simulink model "TosAeb"  (R2021a)
settings: Solver=FixedStepDiscrete  StartTime=0.0  StopTime=inf  FixedStep=0.01  RelTol=1e-3
undefined symbols (not defined in the file or in its folder): ACCModSts, AccSts1, AEB_ObjectMovingOrientation, AEB_ObjectMovingState, CN_BTCActive, CN_CurrentGearR, CN_CurrentGearR_DHT, CN_PTCActive, CN_VDCActive, CN_VSC_EspOff, CN_VSC_FCW_AEB_Error, CN_VSC_FCW_AEB_NoDispay, CN_VSC_FCW_AEB_PerfmDgdtn, CN_VSC_FCW_AEB_TempUnAvlbl, CN_VSC_VehStandstill, CNaccelAvoidMax, CNaccelAvoidMin, CNbrakePedalPress, CNcomfortStrLeftAccelMax, CNcomfortStrLeftAccelMin, CNcomfortStrRightAccelMax, CNcomfortStrRightAccelMin, CNDrvDoorOpen, CNDrvModeEco, CNDrvModeNorm, CNDrvModeOther, CNDrvModeSport, CNDrvSBRreminder, CNNull, CNobjInvalidID, CNPassDoorOpen, CNPassSBRreminder, CNPEBSAccelNegInf, CNPEBSAccelNull, CNPEBSTimeInf, CNPEBSTimeNull, CNSTOPMAX, CNSTOPNULL, CNsubjWidth, CNSwtOFF, CNSwtON, CNTTCMAX, CNTTCNULL, CNVehConfigAEBCycNone, CNVehConfigAEBNone, CNVehConfigAEBPedNone, CNVehConfigFCWNone, CNVehConfigJANone, CollisionSeverity, FALSE, FCWSnvty, JACycObjCalcInfoDefaultParams, JAPedObjCalcInfoDefaultParams, JAVehObjCalcInfoDefaultParams, latCycObjCalcInfoDefaultParams, latPedObjCalcInfoDefaultParams, longObjCalcInfoDefaultParams, ModSts, movingStatus, ObjectType ... (+1112)
libraries: simulink x3031, AEBSFunctionLib x50, Ta_Library x33, AEBSStateMachineLib x23, Mylibrary x12, simulink_extras x7, ObjSelect_lib x3, dspstat3 x2
(part 29 of 102)

== system TosAeb/FcwAebJa_Step_sys/ALG_AEBSystem/ALG_AEBController/AEB_FuncImpManagementApp/FuncManeuArbCalc/brkFuncManeuArbCalc/axTgtReqValueCalc1 ==
  AEBModStsFuncImpArb : Inport
  FCTB_BrkgReqVal : Inport  Port=2
  VehicleStandstill : Inport  Port=3
  VSC_VehStandstill : Inport  Port=4
  subjVel : Inport  Port=5
  subjLongAccel : Inport  Port=6
  ObjectMovingState : Inport  Port=7
  vx : Inport  Port=8
  ax : Inport  Port=9
  dxFrontBumper : Inport  Port=10
  axAbsObj : Inport  Port=11  OutDataTypeStr=single
  vxAbsObj : Inport  Port=12
  AebfullTriggerInfoCalc : Subsystem
  Bus Creator : BusCreator  Inputs=12
  Bus Selector : BusSelector  OutputSignals=AEBModStsFuncImpArb.AEBfullFuncImpArb
  Bus Selector1 : BusSelector  OutputSignals=AEBModStsFuncImpArb.FCTBFuncImpArb,VehicleStandstill,subjVel,FCTB_BrkgReqVal
  Bus Selector2 : BusSelector  OutputSignals=AEBModStsFuncImpArb.AEBfullFuncImpArb,VSC_VehStandstill,subjVel,dxFrontBumper,vx,vxAbsObj,axAbsObj,ax,ObjectMovingState
  Bus Selector3 : BusSelector  OutputSignals=AEBModStsFuncImpArb,subjVel,FCTB_BrkgReqVal,ObjectMovingState
  Bus Selector4 : BusSelector  OutputSignals=VSC_VehStandstill,subjVel,vx,subjLongAccel
  Compare To Constant : simulink/Logic and Bit Operations/Compare To Constant  relop===  const=ModSts.ActiveState  OutDataTypeStr=boolean
  Constant : Constant  Value=P_AEB_FIM_BrkControllerConfig
  Fctb_IsNeedBrakeCalc : Subsystem
  If : If  IfExpression=u1
  Logical Operator : Logic  AllPortsSameDT=off  OutDataTypeStr=boolean
  Merge : Merge
  Unit Delay : UnitDelay  SampleTime=-1  HasFrameUpgradeWarning=on
  axTgtReqValueCalc4AebControl : Subsystem
  axTgtReqValueCalc4AebFull : Subsystem
  FctbIsNeedBrake : Outport
  axTgtReqValue : Outport  Port=2
  wires:
  Bus Selector3/4 -> axTgtReqValueCalc4AebFull/5(ObjectMovingState)  "<ObjectMovingState>"
  Logical Operator/1 -> If/1
  Constant/1 -> Logical Operator/2
  Compare To Constant/1 -> Logical Operator/1
  [axTgtReqValueK1] -> axTgtReqValueCalc4AebControl/9(axTgtReqValueK1)
  Bus Selector4/4 -> axTgtReqValueCalc4AebControl/8(subjLongAccel)  "<subjLongAccel>"
  Bus Selector4/3 -> axTgtReqValueCalc4AebControl/7(vx)  "<vx>"
  AebfullTriggerInfoCalc/4(axAbsObjFilt) -> axTgtReqValueCalc4AebControl/4(axAbsObj)
  Bus Selector4/2 -> axTgtReqValueCalc4AebControl/6(subjVel)  "<subjVel>"
  Bus Selector3/3 -> axTgtReqValueCalc4AebFull/4(FCTB_BrkgReqVal)  "<FCTB_BrkgReqVal>"
  Bus Selector3/2 -> axTgtReqValueCalc4AebFull/3(subjVel)  "<subjVel>"
  Bus Selector3/1 -> axTgtReqValueCalc4AebFull/2(AEBModStsFuncImpArb)  "<AEBModStsFuncImpArb>"
  Unit Delay/1 -> axTgtReqValueCalc4AebFull/6(axTgtReqValueK1), [axTgtReqValueK1]
  axTgtReqValueCalc4AebFull/1(axTgtReqValue) -> Merge/2
  axTgtReqValueCalc4AebControl/1(axTgtReqValue) -> Merge/1
  [AebFullBrkInActive] -> axTgtReqValueCalc4AebFull/Action
  If/2 -> [AebFullBrkInActive]
  Bus Selector2/9 -> AebfullTriggerInfoCalc/9(ObjectMovingState)  "<ObjectMovingState>"
  Bus Selector2/8 -> AebfullTriggerInfoCalc/8(ax)  "<ax>"
  Bus Selector2/5 -> AebfullTriggerInfoCalc/5(vx)  "<vx>"
  Bus Selector2/4 -> AebfullTriggerInfoCalc/4(dxFrontBumper)  "<dxFrontBumper>"
  Bus Selector2/3 -> AebfullTriggerInfoCalc/3(subjVel)  "<subjVel>"
  Bus Selector2/1 -> AebfullTriggerInfoCalc/1(AEBfullFuncImpArb)  "<AEBfullFuncImpArb>"
  Bus Selector1/4 -> Fctb_IsNeedBrakeCalc/4(FCTB_BrkgReqVal)  "<FCTB_BrkgReqVal>"
  Bus Selector1/3 -> Fctb_IsNeedBrakeCalc/3(subjVel)  "<subjVel>"
  Bus Selector1/2 -> Fctb_IsNeedBrakeCalc/2(VehicleStandstill)  "<VehicleStandstill>"
  Bus Selector1/1 -> Fctb_IsNeedBrakeCalc/1(FctbReq)  "<FCTBFuncImpArb>"
  subjLongAccel/1 -> Bus Creator/6  "subjLongAccel"
  Bus Creator/1 -> Bus Selector/1, Bus Selector1/1, Bus Selector3/1, Bus Selector4/1, Bus Selector2/1
  axAbsObj/1 -> Bus Creator/11  "axAbsObj"
  dxFrontBumper/1 -> Bus Creator/10  "dxFrontBumper"
  ax/1 -> Bus Creator/9  "ax"
  vx/1 -> Bus Creator/8  "vx"
  ObjectMovingState/1 -> Bus Creator/7  "ObjectMovingState"
  subjVel/1 -> Bus Creator/5  "subjVel"
  VehicleStandstill/1 -> Bus Creator/3  "VehicleStandstill"
  FCTB_BrkgReqVal/1 -> Bus Creator/2  "FCTB_BrkgReqVal"
  AEBModStsFuncImpArb/1 -> Bus Creator/1  "AEBModStsFuncImpArb"
  If/1 -> axTgtReqValueCalc4AebControl/Action
  Bus Selector/1 -> Compare To Constant/1  "<AEBfullFuncImpArb>"
  AebfullTriggerInfoCalc/3(AEB_FIM_BrakeInfo) -> axTgtReqValueCalc4AebControl/3(AEB_FIM_BrakeInfo)
  AebfullTriggerInfoCalc/2(ObjectPredicted) -> axTgtReqValueCalc4AebControl/2(ObjectPredicted)
  AebfullTriggerInfoCalc/1(AebTriggerInfo) -> axTgtReqValueCalc4AebControl/1(AebTriggerInfo)
  Fctb_IsNeedBrakeCalc/1(IsNeedBrake) -> axTgtReqValueCalc4AebFull/1(AEB_FctbIsNeedBrake), FctbIsNeedBrake/1  "AEB_FctbIsNeedBrake"
  Merge/1 -> axTgtReqValue/1, Unit Delay/1
  VSC_VehStandstill/1 -> Bus Creator/4  "VSC_VehStandstill"
  Bus Selector4/1 -> axTgtReqValueCalc4AebControl/5(VSC_VehStandstill)  "<VSC_VehStandstill>"
  vxAbsObj/1 -> Bus Creator/12  "vxAbsObj"
  Bus Selector2/6 -> AebfullTriggerInfoCalc/6(vxAbsObj)  "<vxAbsObj>"
  Bus Selector2/2 -> AebfullTriggerInfoCalc/2(VSC_VehStandstill)  "<VSC_VehStandstill>"
  Bus Selector2/7 -> AebfullTriggerInfoCalc/7(axAbsObj)  "<axAbsObj>"

== system TosAeb/FcwAebJa_Step_sys/ALG_AEBSystem/ALG_AEBController/AEB_FuncImpManagementApp/FuncManeuArbCalc/brkFuncManeuArbCalc/axTgtReqValueManeuCalc ==
  AEBTrigger : Inport
  axTgtReqValue : Inport  Port=2  OutDataTypeStr=single
  AEBQuitFlgJudge : Subsystem
  AEBTriggerManeuCalc : Stateflow chart  TreatAsAtomicUnit=on
  AEBTriggerManeuCalc1 : Stateflow chart  Commented=on  TreatAsAtomicUnit=on
  Constant : Constant  Value=Ts  OutDataTypeStr=single
  Constant1 : Constant  Value=P_AEB_FIM_BrkFuncMaxTimeQuitThres  OutDataTypeStr=single
  Constant2 : Constant  Value=P_AEB_FIM_DecelGradientQuitThres  OutDataTypeStr=single
  Unit Delay1 : UnitDelay  SampleTime=-1  HasFrameUpgradeWarning=on
  AEBTriggerManeu : Outport
  axTgtReqValueManeu : Outport  Port=2
  wires:
  Unit Delay1/1 -> AEBTriggerManeuCalc/7(axTgtReqValueManeuK1)
  AEBTriggerManeuCalc/2(axTgtReqValueManeu) -> axTgtReqValueManeu/1, Unit Delay1/1
  AEBTriggerManeuCalc/1(AEBTriggerManeu) -> AEBTriggerManeu/1
  axTgtReqValue/1 -> AEBTriggerManeuCalc/3(axTgtReqValue)
  Constant/1 -> AEBTriggerManeuCalc/4(Ts)
  Constant1/1 -> AEBTriggerManeuCalc/5(PmaxQuitTime)
  AEBQuitFlgJudge/1(AEBQuitFlg) -> AEBTriggerManeuCalc/1(AEBQuitFlg)
  AEBTrigger/1 -> AEBQuitFlgJudge/1(AEBTrigger), AEBTriggerManeuCalc/2(AEBTrigger)
  Constant2/1 -> AEBTriggerManeuCalc/6(axJerkQuit)

== system TosAeb/FcwAebJa_Step_sys/ALG_AEBSystem/ALG_AEBController/AEB_FuncImpManagementApp/FuncManeuArbCalc/brkFuncManeuArbCalc/brkManeuAborCalc ==
  AEBTrigger : Inport
  subjVel : Inport  Port=2  OutDataTypeStr=single
  EBAFuncSts : Inport  Port=3
  accelAvoid : Inport  Port=4
  ObjectMovingState : Inport  Port=5
  axAbsObj : Inport  Port=6
  AEBBA : Inport  Port=7
  AEBIB : Inport  Port=8
  vx : Inport  Port=9
  Bus Creator : BusCreator  Inputs=5
  Bus Selector : BusSelector  OutputSignals=AEBTrigger,VelReduceMaxCond,dgrCond,ESPRelAborCond,VelCond
  Bus Selector1 : BusSelector  OutputSignals=AEBTrigger,VelReduceMaxCond,dgrCond,ESPRelAborCond
  Bus Selector2 : BusSelector  OutputSignals=AEBTrigger,VelReduceMaxCond,dgrCond,ESPRelAborCond
  Constant : Constant  Value=FALSE
  Constant1 : Constant  Value=FALSE
  Constant2 : Constant  Value=FALSE
  DgrCondCalc : Subsystem
  ESPRelAborCondCalc : Subsystem
  JABrkManeuAborCalc : Subsystem
  LatBrkManeuAborCalc : Subsystem
  Terminator : Terminator
  Terminator1 : Terminator
  Terminator2 : Terminator
  VelCond : Subsystem
  VelReduceMaxCond : Subsystem
  vehBrkManeuAborCalc : Subsystem
  vehBrkManeuAbor : Outport
  latBrkManeuAbor : Outport  Port=2
  JABrkManeuAbor : Outport  Port=3
  wires:
  Bus Selector/5 -> vehBrkManeuAborCalc/5(velAborCond)  "<VelCond>"
  VelCond/1(VelCond) -> Bus Creator/3  "VelCond"
  vx/1 -> VelCond/1(vx)
  EBAFuncSts/1 -> VelReduceMaxCond/3(EBAFuncSts)
  JABrkManeuAborCalc/1(JABrkManeuAbor) -> JABrkManeuAbor/1
  LatBrkManeuAborCalc/1(latBrkManeuAbor) -> latBrkManeuAbor/1
  Constant2/1 -> JABrkManeuAborCalc/2(VelReduceMaxCond)
  Bus Selector2/2 -> Terminator2/1  "<VelReduceMaxCond>"
  Bus Selector2/1 -> JABrkManeuAborCalc/1(AEBTrigger)  "<AEBTrigger>"
  Bus Selector2/3 -> Terminator1/1  "<dgrCond>"
  Constant1/1 -> JABrkManeuAborCalc/3(dgrAborCond)
  Bus Selector2/4 -> JABrkManeuAborCalc/4(ESPRelAborCond)  "<ESPRelAborCond>"
  Bus Selector1/4 -> LatBrkManeuAborCalc/4(ESPRelAborCond)  "<ESPRelAborCond>"
  Constant/1 -> LatBrkManeuAborCalc/3(dgrAborCond)
  Bus Selector1/3 -> Terminator/1  "<dgrCond>"
  Bus Selector1/2 -> LatBrkManeuAborCalc/2(VelReduceMaxCond)  "<VelReduceMaxCond>"
  Bus Selector1/1 -> LatBrkManeuAborCalc/1(AEBTrigger)  "<AEBTrigger>"
  Bus Selector/1 -> vehBrkManeuAborCalc/1(AEBTrigger)  "<AEBTrigger>"
  Bus Selector/4 -> vehBrkManeuAborCalc/4(ESPRelAborCond)  "<ESPRelAborCond>"
  Bus Selector/2 -> vehBrkManeuAborCalc/2(VelReduceMaxCond)  "<VelReduceMaxCond>"
  Bus Selector/3 -> vehBrkManeuAborCalc/3(dgrAborCond)  "<dgrCond>"
  Bus Creator/1 -> Bus Selector1/1, Bus Selector2/1, Bus Selector/1
  ESPRelAborCondCalc/1(ESPRelAborCond) -> Bus Creator/5  "ESPRelAborCond"
  DgrCondCalc/1(dgrCond) -> Bus Creator/4  "dgrCond"
  VelReduceMaxCond/1(VelReduceMaxCond) -> Bus Creator/2  "VelReduceMaxCond"
  AEBIB/1 -> ESPRelAborCondCalc/2(AEBIB)
  AEBBA/1 -> ESPRelAborCondCalc/1(AEBBA)
  accelAvoid/1 -> DgrCondCalc/3(accelAvoid)
  ObjectMovingState/1 -> DgrCondCalc/1(ObjectMovingState), VelCond/3(ObjectMovingState)
  axAbsObj/1 -> DgrCondCalc/2(axAbsObj), VelCond/2(axAbsObj)
  vehBrkManeuAborCalc/1(vehBrkManeuAbor) -> vehBrkManeuAbor/1
  AEBTrigger/1 -> VelReduceMaxCond/1(AEBTrigger), Bus Creator/1  "AEBTrigger"
  subjVel/1 -> VelReduceMaxCond/2(subjVel)

== system TosAeb/FcwAebJa_Step_sys/ALG_AEBSystem/ALG_AEBController/AEB_JaObjCalcApp/JAPedTgtInfoCalc/JAPedCollisonAssessInfo/LatPedCollisonAssessInfoCalc ==
  vx : Inport  OutDataTypeStr=single
  vy : Inport  Port=2  OutDataTypeStr=single
  dx : Inport  Port=3  OutDataTypeStr=single
  dy : Inport  Port=4  OutDataTypeStr=single
  dxFrontBumper : Inport  Port=5  OutDataTypeStr=single
  tPredictTime : Inport  Port=6  OutDataTypeStr=single
  subjYawRate : Inport  Port=7  OutDataTypeStr=single
  subjVel1 : Inport  Port=8  OutDataTypeStr=single
  subjLongAccel : Inport  Port=9  OutDataTypeStr=single
  Action Port : ActionPort  ActionPortLabel=else
  Bus Creator : BusCreator  Inputs=4
  PedDistBrakeFullCalc : Subsystem
  Subsystem1 : Subsystem
  VelAbsTgtCorrCalc : Subsystem
  distRadical2CollsionCalc : Subsystem
  tBrakeingCalc : Subsystem  Variant=on
  JAPedCollisonAssessInfo : Outport
  wires:
  Bus Creator/1 -> JAPedCollisonAssessInfo/1
  VelAbsTgtCorrCalc/2(vyAbsTgtCorr) -> distRadical2CollsionCalc/2(vyAbsTgtCorr), Subsystem1/2(vyAbsTargetCorr)
  tPredictTime/1 -> distRadical2CollsionCalc/5(tPredictTime)
  tBrakeingCalc/1(TTB) -> Bus Creator/4  "TTB"
  subjLongAccel/1 -> PedDistBrakeFullCalc/1(subjLongAccel)
  dxFrontBumper/1 -> VelAbsTgtCorrCalc/4(dxFrontBumper)
  Subsystem1/1(TgtYawAngle) -> Bus Creator/1  "TgtYawAngle"
  subjYawRate/1 -> VelAbsTgtCorrCalc/5(subjYawRate)
  PedDistBrakeFullCalc/1(distBrake) -> Bus Creator/3  "distBraking"
  [dy] -> distRadical2CollsionCalc/4(dy)
  vy/1 -> VelAbsTgtCorrCalc/2(vy)
  dx/1 -> distRadical2CollsionCalc/3(dx)
  PedDistBrakeFullCalc/2(tBrake) -> tBrakeingCalc/1(tBarke)
  dy/1 -> [dy], VelAbsTgtCorrCalc/3(dy)
  distRadical2CollsionCalc/1(distRadical2Collsion) -> Bus Creator/2  "distRadical2Collsion"
  subjVel1/1 -> VelAbsTgtCorrCalc/6(subjVel), PedDistBrakeFullCalc/2(subjVel), tBrakeingCalc/2(subjVel)
  VelAbsTgtCorrCalc/1(vxAbsTgtCorr) -> distRadical2CollsionCalc/1(vxAbsTgtCorr), Subsystem1/1(vxAbsTargetCorr)
  vx/1 -> VelAbsTgtCorrCalc/1(vx)

== system TosAeb/FcwAebJa_Step_sys/ALG_AEBSystem/ALG_AEBController/AEB_JaObjCalcApp/JAPedTgtInfoCalc/JAPedCollisonAssessInfo/LatPedCollisonAssessInfoDefault ==
  Action Port : ActionPort  ActionPortLabel=if(u1)
  Bus Creator : BusCreator  Inputs=4
  Constant : Constant  Value=0  OutDataTypeStr=single
  Constant1 : Constant  Value=255  OutDataTypeStr=single
  Constant2 : Constant  Value=255  OutDataTypeStr=single
  Constant3 : Constant  Value=256  OutDataTypeStr=single
  JAPedCollisonAssessInfo : Outport
  wires:
  Constant2/1 -> Bus Creator/3  "distBraking"
  Constant1/1 -> Bus Creator/2  "distRadical2Collsion"
  Bus Creator/1 -> JAPedCollisonAssessInfo/1
  Constant/1 -> Bus Creator/1  "TgtYawAngle"
  Constant3/1 -> Bus Creator/4  "TTB"

== system TosAeb/FcwAebJa_Step_sys/ALG_AEBSystem/ALG_AEBController/AEB_JaObjCalcApp/JAPedTgtInfoCalc/JAPedCollisonAssessInfo/ObjIsInvalidJudge ==
  ObjectID : Inport
  Compare To Constant : simulink/Logic and Bit Operations/Compare To Constant  relop===  const=CNobjInvalidID  OutDataTypeStr=boolean
  ObjIsInvalid : Outport
  wires:
  ObjectID/1 -> Compare To Constant/1
  Compare To Constant/1 -> ObjIsInvalid/1

== system TosAeb/FcwAebJa_Step_sys/ALG_AEBSystem/ALG_AEBController/AEB_JaObjCalcApp/JAPedTgtInfoCalc/JAPedObjCalcInfoCalc/JAPedObjInfoCalc ==
  AEBJAVehObj : Inport
  subjInfo : Inport  Port=2
  Action Port : ActionPort  ActionPortLabel=else
  Bus Creator : BusCreator  Inputs=3  OutDataTypeStr=Bus: BusAEBJAPedObjCalcInfo  NonVirtualBus=on
      settings: InheritFromInputs=off
  Bus Creator1 : BusCreator  Inputs=6  OutDataTypeStr=Bus: BusAEBJAPedObjAbsInfo  NonVirtualBus=on
      settings: InheritFromInputs=off
  Bus Selector : BusSelector  OutputSignals=dx,dy,vx,ax,ObjectID,ObjectTypeCameraConfirmed,FR_ObjectID,FR_Dx,FR_Vy,FC_ObjectID,FC_Dx,FC_Vy
  Bus Selector1 : BusSelector  OutputSignals=subjLongAccel,subjYawRate,subjVel
  ObjCrossFlgCal : Subsystem
  SensorTgtInfo2AbsTgtInfoTrans : Subsystem
  "JAPedObjCalcInfo " : Outport  OutDataTypeStr=Bus: BusAEBJAPedObjCalcInfo
  wires:
  ObjCrossFlgCal/1(ObjColliInfo) -> Bus Creator/3  "BusAEBJAObjColliInfo"
  [ObjAbsInfo] -> Bus Creator/2  "BusAEBJAObjAbsInfo"
  Bus Creator1/1 -> [ObjAbsInfo], ObjCrossFlgCal/2(ObjAbsInfo)
  subjInfo/1 -> Bus Selector1/1, ObjCrossFlgCal/3(subjInfo)
  AEBJAVehObj/1 -> Bus Selector/1, Bus Creator/1, ObjCrossFlgCal/1(AEBJAPedObj)  "AEBJAPedObj"
  Bus Creator/1 -> "JAPedObjCalcInfo "/1
  SensorTgtInfo2AbsTgtInfoTrans/6(ObjectYawAngleCorr) -> Bus Creator1/6  "ObjectYawAngleCorr"
  Bus Selector/7 -> SensorTgtInfo2AbsTgtInfoTrans/7(FR_ObjectID)  "<FR_ObjectID>"
  SensorTgtInfo2AbsTgtInfoTrans/5(ObjFusQuality) -> Bus Creator1/5  "ObjFusQuality"
  SensorTgtInfo2AbsTgtInfoTrans/1(dxFrontBumper) -> Bus Creator1/1  "dxFrontBumper"
  Bus Selector1/1 -> SensorTgtInfo2AbsTgtInfoTrans/13(subjLongAccel)  "<subjLongAccel>"
  Bus Selector/1 -> SensorTgtInfo2AbsTgtInfoTrans/1(dx)  "<dx>"
  Bus Selector/5 -> SensorTgtInfo2AbsTgtInfoTrans/5(ObjectID)  "<ObjectID>"
  SensorTgtInfo2AbsTgtInfoTrans/2(vxAbsObj) -> Bus Creator1/2  "vxAbsObj"
  Bus Selector1/3 -> SensorTgtInfo2AbsTgtInfoTrans/15(subjVel)  "<subjVel>"
  Bus Selector/10 -> SensorTgtInfo2AbsTgtInfoTrans/10(FC_ObjectID)  "<FC_ObjectID>"
  Bus Selector/4 -> SensorTgtInfo2AbsTgtInfoTrans/4(ax)  "<ax>"
  Bus Selector/12 -> SensorTgtInfo2AbsTgtInfoTrans/12(FC_Vy)  "<FC_Vy>"
  Bus Selector/11 -> SensorTgtInfo2AbsTgtInfoTrans/11(FC_Dx)  "<FC_Dx>"
  Bus Selector1/2 -> SensorTgtInfo2AbsTgtInfoTrans/14(subjYawRate)  "<subjYawRate>"
  Bus Selector/3 -> SensorTgtInfo2AbsTgtInfoTrans/3(vx)  "<vx>"
  Bus Selector/2 -> SensorTgtInfo2AbsTgtInfoTrans/2(dy)  "<dy>"
  SensorTgtInfo2AbsTgtInfoTrans/3(axAbsObj) -> Bus Creator1/3  "axAbsObj"
  Bus Selector/6 -> SensorTgtInfo2AbsTgtInfoTrans/6(ObjectTypeCameraConfirmed)  "<ObjectTypeCameraConfirmed>"
  SensorTgtInfo2AbsTgtInfoTrans/4(vyRelCorr) -> Bus Creator1/4  "vyRelCorr"
  Bus Selector/9 -> SensorTgtInfo2AbsTgtInfoTrans/9(FR_Vy)  "<FR_Vy>"
  Bus Selector/8 -> SensorTgtInfo2AbsTgtInfoTrans/8(FR_Dx)  "<FR_Dx>"

== system TosAeb/FcwAebJa_Step_sys/ALG_AEBSystem/ALG_AEBController/AEB_JaObjCalcApp/JAPedTgtInfoCalc/JAPedObjCalcInfoCalc/JAPedObjInfoDefault ==
  Action Port : ActionPort  ActionPortLabel=if(u1)
  Constant : Constant  Value=JAPedObjCalcInfoDefaultParams  OutDataTypeStr=Bus: BusAEBJAPedObjCalcInfo
  JAPedObjCalcInfo : Outport  OutDataTypeStr=Bus: BusAEBJAPedObjCalcInfo
  wires:
  Constant/1 -> JAPedObjCalcInfo/1

== system TosAeb/FcwAebJa_Step_sys/ALG_AEBSystem/ALG_AEBController/AEB_JaObjCalcApp/JAPedTgtInfoCalc/JAPedObjCalcInfoCalc/ObjPreSelectCondCalc ==
  BusAEBJaObj : Inport  OutDataTypeStr=Bus: BusAEBJAObjBus
  Bus Selector : BusSelector  OutputSignals=ObjectID,ObjectExistProb,ObjectYawAngle,ObjectTypeCameraConfirmed,ObjectType,dxVariance,dx,dy
  If : If  IfExpression=u1
  Merge : Merge
  ObjIsInvalidCalc : Subsystem
  TgtPreSelectCondCalc : Subsystem
  TgtPreSelectCondCalc1 : Subsystem  TreatAsAtomicUnit=on
  ObjPreSelectCond : Outport  OutDataTypeStr=boolean  LockScale=on
  wires:
  BusAEBJaObj/1 -> Bus Selector/1
  Bus Selector/1 -> ObjIsInvalidCalc/1(ObjectID)  "<ObjectID>"
  Bus Selector/2 -> TgtPreSelectCondCalc/1(ObjectExistProb)  "<ObjectExistProb>"
  Bus Selector/3 -> TgtPreSelectCondCalc/2(ObjectYawAngle)  "<ObjectYawAngle>"
  Bus Selector/4 -> TgtPreSelectCondCalc/3(ObjectTypeCameraComfirmed)  "<ObjectTypeCameraConfirmed>"
  Bus Selector/5 -> TgtPreSelectCondCalc/4(ObjectType)  "<ObjectType>"
  Bus Selector/6 -> TgtPreSelectCondCalc/5(dxVariance)  "<dxVariance>"
  Bus Selector/7 -> TgtPreSelectCondCalc/6(dx)  "<dx>"
  Bus Selector/8 -> TgtPreSelectCondCalc/7(dy)  "<dy>"
  TgtPreSelectCondCalc1/1(ObjPreSelectCondFufilled) -> Merge/1
  TgtPreSelectCondCalc/1(ObjPreSelectCondFufilled) -> Merge/2
  If/2 -> TgtPreSelectCondCalc/Action
  If/1 -> TgtPreSelectCondCalc1/Action
  ObjIsInvalidCalc/1(ObjIsInvalid) -> If/1
  Merge/1 -> ObjPreSelectCond/1

== system TosAeb/FcwAebJa_Step_sys/ALG_AEBSystem/ALG_AEBController/AEB_JaObjCalcApp/JAPedTgtInfoCalc/TargetSelectCalc/tgtSelectFlow ==
  JAPedObjCalcInfo : Inport
  ObjPreSelectCondFufilled : Inport  Port=2
  subjVel : Inport  Port=3
  SteerWheelAngle_Deg : Inport  Port=4
  JAPedPrefillModSts : Inport  Port=5
  JAPedAEBfullModSts : Inport  Port=6
  Bus Selector : BusSelector  OutputSignals=AEBJAPedObj.ObjectID
  Constant : Constant  Value=P_AEB_JaPedCA_SelectStrWheelAngleAbsThres
  Constant1 : Constant  Value=P_AEB_JaPedCA_SelectSubjVelThres
  Unit Delay : UnitDelay  InitialCondition=1  SampleTime=-1  HasFrameUpgradeWarning=on
  Unit Delay1 : UnitDelay  SampleTime=-1  HasFrameUpgradeWarning=on
  tgtObjFlow : Stateflow chart  TreatAsAtomicUnit=on
  JAPedTgtObjInfo : Outport
  wires:
  JAPedObjCalcInfo/1 -> tgtObjFlow/1(JaPedObjCalcInfo)
  SteerWheelAngle_Deg/1 -> tgtObjFlow/4(SteerWheelAngle_Deg)
  Unit Delay1/1 -> tgtObjFlow/7(TargetIDK1)
  Bus Selector/1 -> Unit Delay1/1  "<ObjectID>"
  JAPedPrefillModSts/1 -> tgtObjFlow/5(JAPedAEBfullModSts)
  tgtObjFlow/2(tgtIndex) -> Unit Delay/1
  Unit Delay/1 -> tgtObjFlow/8(tgtIndexK1)
  ObjPreSelectCondFufilled/1 -> tgtObjFlow/2(ObjPreSelectCondFufilled)
  subjVel/1 -> tgtObjFlow/3(subjVel)
  JAPedAEBfullModSts/1 -> tgtObjFlow/6(JAPedPrefillModSts)
  tgtObjFlow/1(JaPedTgtObjInfo) -> Bus Selector/1, JAPedTgtObjInfo/1  "JAPedTgtObjInfo"
  Constant/1 -> tgtObjFlow/9(P_JaPedCA_SelectStrWheelAngleAbsThres)
  Constant1/1 -> tgtObjFlow/10(P_JaPedCA_SelectSubjVelThres)

== system TosAeb/FcwAebJa_Step_sys/ALG_AEBSystem/ALG_AEBController/AEB_JaObjCalcApp/JAVehTgtInfoCalc/AEBJAVehObjCalc/JAVehObjCalcInfoDefault ==
  Action Port : ActionPort  ActionPortLabel=if(u1)
  JAVehObjCalcInfoDefaultParams : Constant  Value=JAVehObjCalcInfoDefaultParams  OutDataTypeStr=Bus: BusAEBJAVehObjCalcInfo
  JAVehObjCalcInfo : Outport
  wires:
  JAVehObjCalcInfoDefaultParams/1 -> JAVehObjCalcInfo/1

== system TosAeb/FcwAebJa_Step_sys/ALG_AEBSystem/ALG_AEBController/AEB_JaObjCalcApp/JAVehTgtInfoCalc/AEBJAVehObjCalc/JAVehObjInfoCalc ==
  subjInfo : Inport
  AEBJAVehObj : Inport  Port=2
  Action Port : ActionPort  ActionPortLabel=else
  Bus Creator : BusCreator  Inputs=3  OutDataTypeStr=Bus: BusAEBJAVehObjCalcInfo  NonVirtualBus=on
      settings: InheritFromInputs=off
  Bus Creator1 : BusCreator  Inputs=5  OutDataTypeStr=Bus: BusAEBJAVehObjAbsInfo  NonVirtualBus=on
      settings: InheritFromInputs=off
  Bus Selector : BusSelector  OutputSignals=dx,vx,ax,vy,ObjectID,ObjectTypeCameraConfirmed,FR_ObjectID,FR_Dx,FR_Dy,FC_ObjectID,FC_Dx,FC_Dy
  Bus Selector1 : BusSelector  OutputSignals=subjVel,subjLongAccel,subjYawRate
  ObjCrossFlgCal : Subsystem
  SensorTgtInfo2AbsTgtInfoTrans : Subsystem
  JAVehObjCalcInfo : Outport  OutDataTypeStr=Bus: BusAEBJAVehObjCalcInfo
  wires:
  Bus Selector/1 -> SensorTgtInfo2AbsTgtInfoTrans/1(dx)  "<dx>"
  Bus Selector1/1 -> SensorTgtInfo2AbsTgtInfoTrans/13(subjVel)  "<subjVel>"
  Bus Selector/4 -> SensorTgtInfo2AbsTgtInfoTrans/4(vy)  "<vy>"
  Bus Selector1/3 -> SensorTgtInfo2AbsTgtInfoTrans/15(subjYawRate)  "<subjYawRate>"
  Bus Creator1/1 -> [ObjAbsInfo], ObjCrossFlgCal/2(ObjAbsInfo)
  Bus Selector1/2 -> SensorTgtInfo2AbsTgtInfoTrans/14(subjLongAccel)  "<subjLongAccel>"
  AEBJAVehObj/1 -> Bus Selector/1, ObjCrossFlgCal/1(BusAEBJAVehObj), Bus Creator/1
  Bus Selector/2 -> SensorTgtInfo2AbsTgtInfoTrans/2(vx)  "<vx>"
  subjInfo/1 -> ObjCrossFlgCal/3(subjInfo), Bus Selector1/1
  SensorTgtInfo2AbsTgtInfoTrans/1(dxFrontBumper) -> Bus Creator1/1  "dxFrontBumper"
  SensorTgtInfo2AbsTgtInfoTrans/3(axAbsObj) -> Bus Creator1/3  "axAbsObj"
  SensorTgtInfo2AbsTgtInfoTrans/2(vxAbsObj) -> Bus Creator1/2  "vxAbsObj"
  Bus Selector/3 -> SensorTgtInfo2AbsTgtInfoTrans/3(ax)  "<ax>"
  Bus Creator/1 -> JAVehObjCalcInfo/1
  [ObjAbsInfo] -> Bus Creator/2  "BusAEBJAObjAbsInfo"
  ObjCrossFlgCal/1(ObjColliInfo) -> Bus Creator/3  "BusAEBJAObjColliInfo"
  SensorTgtInfo2AbsTgtInfoTrans/4(vyRelCorr) -> Bus Creator1/4  "vyRelCorr"
  SensorTgtInfo2AbsTgtInfoTrans/5(ObjFusQuality) -> Bus Creator1/5  "ObjFusQuality"
  Bus Selector/5 -> SensorTgtInfo2AbsTgtInfoTrans/5(ObjectID)  "<ObjectID>"
  Bus Selector/6 -> SensorTgtInfo2AbsTgtInfoTrans/6(ObjectTypeCameraConfirmed)  "<ObjectTypeCameraConfirmed>"
  Bus Selector/7 -> SensorTgtInfo2AbsTgtInfoTrans/7(FR_ObjectID)  "<FR_ObjectID>"
  Bus Selector/8 -> SensorTgtInfo2AbsTgtInfoTrans/8(FR_Dx)  "<FR_Dx>"
  Bus Selector/9 -> SensorTgtInfo2AbsTgtInfoTrans/9(FR_Dy)  "<FR_Dy>"
  Bus Selector/10 -> SensorTgtInfo2AbsTgtInfoTrans/10(FC_ObjectID)  "<FC_ObjectID>"
  Bus Selector/11 -> SensorTgtInfo2AbsTgtInfoTrans/11(FC_Dx)  "<FC_Dx>"
  Bus Selector/12 -> SensorTgtInfo2AbsTgtInfoTrans/12(FC_Dy)  "<FC_Dy>"
